simulink model "dss_ideal_8192ch_1966mhz_cx"  (R2021a) (continued)
== system dss_ideal_8192ch_1966mhz_cx == (continued)
      mask help: This simple vector accumulator is based on a shift register. It outputs its previous accumulated vector after a new_acc pulse is received.
      mask init:
      | simple_bram_vacc_init(gcb, ...
      |     'vec_len', vec_len, ...
      |     'arith_type', arith_type, ...
      |     'n_bits', n_bits, ...
      |     'bin_pt', bin_pt);
      mask TypeOptions arith_type: Signed | Unsigned
  simple_bram_vacc28 : Subsystem  AncestorBlock=casper_library_accumulators/simple_bram_vacc  mask(simple_bram_vacc){vec_len=1024, arith_type=Unsigned, n_bits=64, bin_pt=36}
      mask prompts: vec_len "Vector length:"; arith_type "Output type:"; n_bits "Bit width (output):"; bin_pt "Binary point (output):"
      mask help: This simple vector accumulator is based on a shift register. It outputs its previous accumulated vector after a new_acc pulse is received.
      mask init:
      | simple_bram_vacc_init(gcb, ...
      |     'vec_len', vec_len, ...
      |     'arith_type', arith_type, ...
      |     'n_bits', n_bits, ...
      |     'bin_pt', bin_pt);
      mask TypeOptions arith_type: Signed | Unsigned
  simple_bram_vacc29 : Subsystem  AncestorBlock=casper_library_accumulators/simple_bram_vacc  mask(simple_bram_vacc){vec_len=1024, arith_type=Unsigned, n_bits=64, bin_pt=36}
      mask prompts: vec_len "Vector length:"; arith_type "Output type:"; n_bits "Bit width (output):"; bin_pt "Binary point (output):"
      mask help: This simple vector accumulator is based on a shift register. It outputs its previous accumulated vector after a new_acc pulse is received.
      mask init:
      | simple_bram_vacc_init(gcb, ...
      |     'vec_len', vec_len, ...
      |     'arith_type', arith_type, ...
      |     'n_bits', n_bits, ...
      |     'bin_pt', bin_pt);
      mask TypeOptions arith_type: Signed | Unsigned
  simple_bram_vacc3 : Subsystem  AncestorBlock=casper_library_accumulators/simple_bram_vacc  mask(simple_bram_vacc){vec_len=1024, arith_type=Unsigned, n_bits=64, bin_pt=36}
      mask prompts: vec_len "Vector length:"; arith_type "Output type:"; n_bits "Bit width (output):"; bin_pt "Binary point (output):"
      mask help: This simple vector accumulator is based on a shift register. It outputs its previous accumulated vector after a new_acc pulse is received.
      mask init:
      | simple_bram_vacc_init(gcb, ...
      |     'vec_len', vec_len, ...
      |     'arith_type', arith_type, ...
      |     'n_bits', n_bits, ...
      |     'bin_pt', bin_pt);
      mask TypeOptions arith_type: Signed | Unsigned
  simple_bram_vacc30 : Subsystem  AncestorBlock=casper_library_accumulators/simple_bram_vacc  mask(simple_bram_vacc){vec_len=1024, arith_type=Unsigned, n_bits=64, bin_pt=36}
      mask prompts: vec_len "Vector length:"; arith_type "Output type:"; n_bits "Bit width (output):"; bin_pt "Binary point (output):"
      mask help: This simple vector accumulator is based on a shift register. It outputs its previous accumulated vector after a new_acc pulse is received.
      mask init:
      | simple_bram_vacc_init(gcb, ...
      |     'vec_len', vec_len, ...
      |     'arith_type', arith_type, ...
      |     'n_bits', n_bits, ...
      |     'bin_pt', bin_pt);
      mask TypeOptions arith_type: Signed | Unsigned
  simple_bram_vacc31 : Subsystem  AncestorBlock=casper_library_accumulators/simple_bram_vacc  mask(simple_bram_vacc){vec_len=1024, arith_type=Unsigned, n_bits=64, bin_pt=36}
      mask prompts: vec_len "Vector length:"; arith_type "Output type:"; n_bits "Bit width (output):"; bin_pt "Binary point (output):"
      mask help: This simple vector accumulator is based on a shift register. It outputs its previous accumulated vector after a new_acc pulse is received.
      mask init:
      | simple_bram_vacc_init(gcb, ...
      |     'vec_len', vec_len, ...
      |     'arith_type', arith_type, ...
      |     'n_bits', n_bits, ...
      |     'bin_pt', bin_pt);
      mask TypeOptions arith_type: Signed | Unsigned
  simple_bram_vacc32 : Subsystem  AncestorBlock=casper_library_accumulators/simple_bram_vacc  mask(simple_bram_vacc){vec_len=1024, arith_type=Unsigned, n_bits=64, bin_pt=36}
      mask prompts: vec_len "Vector length:"; arith_type "Output type:"; n_bits "Bit width (output):"; bin_pt "Binary point (output):"
      mask help: This simple vector accumulator is based on a shift register. It outputs its previous accumulated vector after a new_acc pulse is received.
      mask init:
      | simple_bram_vacc_init(gcb, ...
      |     'vec_len', vec_len, ...
      |     'arith_type', arith_type, ...
      |     'n_bits', n_bits, ...
      |     'bin_pt', bin_pt);
      mask TypeOptions arith_type: Signed | Unsigned
  simple_bram_vacc4 : Subsystem  AncestorBlock=casper_library_accumulators/simple_bram_vacc  mask(simple_bram_vacc){vec_len=1024, arith_type=Unsigned, n_bits=64, bin_pt=36}
      mask prompts: vec_len "Vector length:"; arith_type "Output type:"; n_bits "Bit width (output):"; bin_pt "Binary point (output):"
      mask help: This simple vector accumulator is based on a shift register. It outputs its previous accumulated vector after a new_acc pulse is received.
      mask init:
      | simple_bram_vacc_init(gcb, ...
      |     'vec_len', vec_len, ...
      |     'arith_type', arith_type, ...
      |     'n_bits', n_bits, ...
      |     'bin_pt', bin_pt);
      mask TypeOptions arith_type: Signed | Unsigned
  simple_bram_vacc5 : Subsystem  AncestorBlock=casper_library_accumulators/simple_bram_vacc  mask(simple_bram_vacc){vec_len=1024, arith_type=Unsigned, n_bits=64, bin_pt=36}
      mask prompts: vec_len "Vector length:"; arith_type "Output type:"; n_bits "Bit width (output):"; bin_pt "Binary point (output):"
      mask help: This simple vector accumulator is based on a shift register. It outputs its previous accumulated vector after a new_acc pulse is received.
      mask init:
      | simple_bram_vacc_init(gcb, ...
      |     'vec_len', vec_len, ...
      |     'arith_type', arith_type, ...
      |     'n_bits', n_bits, ...
      |     'bin_pt', bin_pt);
      mask TypeOptions arith_type: Signed | Unsigned
  simple_bram_vacc6 : Subsystem  AncestorBlock=casper_library_accumulators/simple_bram_vacc  mask(simple_bram_vacc){vec_len=1024, arith_type=Unsigned, n_bits=64, bin_pt=36}
      mask prompts: vec_len "Vector length:"; arith_type "Output type:"; n_bits "Bit width (output):"; bin_pt "Binary point (output):"
      mask help: This simple vector accumulator is based on a shift register. It outputs its previous accumulated vector after a new_acc pulse is received.
      mask init:
      | simple_bram_vacc_init(gcb, ...
      |     'vec_len', vec_len, ...
      |     'arith_type', arith_type, ...
      |     'n_bits', n_bits, ...
      |     'bin_pt', bin_pt);
      mask TypeOptions arith_type: Signed | Unsigned
  simple_bram_vacc7 : Subsystem  AncestorBlock=casper_library_accumulators/simple_bram_vacc  mask(simple_bram_vacc){vec_len=1024, arith_type=Unsigned, n_bits=64, bin_pt=36}
      mask prompts: vec_len "Vector length:"; arith_type "Output type:"; n_bits "Bit width (output):"; bin_pt "Binary point (output):"
      mask help: This simple vector accumulator is based on a shift register. It outputs its previous accumulated vector after a new_acc pulse is received.
      mask init:
      | simple_bram_vacc_init(gcb, ...
      |     'vec_len', vec_len, ...
      |     'arith_type', arith_type, ...
      |     'n_bits', n_bits, ...
      |     'bin_pt', bin_pt);
      mask TypeOptions arith_type: Signed | Unsigned
  simple_bram_vacc8 : Subsystem  AncestorBlock=casper_library_accumulators/simple_bram_vacc  mask(simple_bram_vacc){vec_len=1024, arith_type=Unsigned, n_bits=64, bin_pt=36}
      mask prompts: vec_len "Vector length:"; arith_type "Output type:"; n_bits "Bit width (output):"; bin_pt "Binary point (output):"
      mask help: This simple vector accumulator is based on a shift register. It outputs its previous accumulated vector after a new_acc pulse is received.
      mask init:
      | simple_bram_vacc_init(gcb, ...
      |     'vec_len', vec_len, ...
      |     'arith_type', arith_type, ...
      |     'n_bits', n_bits, ...
      |     'bin_pt', bin_pt);
      mask TypeOptions arith_type: Signed | Unsigned
  simple_bram_vacc9 : Subsystem  AncestorBlock=casper_library_accumulators/simple_bram_vacc  mask(simple_bram_vacc){vec_len=1024, arith_type=Unsigned, n_bits=64, bin_pt=36}
      mask prompts: vec_len "Vector length:"; arith_type "Output type:"; n_bits "Bit width (output):"; bin_pt "Binary point (output):"
      mask help: This simple vector accumulator is based on a shift register. It outputs its previous accumulated vector after a new_acc pulse is received.
      mask init:
      | simple_bram_vacc_init(gcb, ...
      |     'vec_len', vec_len, ...
      |     'arith_type', arith_type, ...
      |     'n_bits', n_bits, ...
      |     'bin_pt', bin_pt);
      mask TypeOptions arith_type: Signed | Unsigned
  spect_cnt2 : xbsIndex_r4/Counter  cnt_type=Free Running  cnt_to=Inf  operation=Up  start_count=0  cnt_by_val=1  arith_type=Unsigned  n_bits=10  bin_pt=0  load_pin=off  rst=on  en=on  explicit_period=on  period=1  use_behavioral_HDL=off  implementation=Fabric  use_rpm=off  dbl_ovrd=off  block_type=counter
  sync_gen : Subsystem  AncestorBlock=casper_library_misc/sync_gen  mask(sync_gen){sim_acc_len=1, fft_size=8192, fft_simult_inputs=8, pfb_fir_taps=4, reorder_vec=[2 2 10], scale=8192}
      mask prompts: sim_acc_len "Simulation Accumulation Length"; fft_size "FFT Size"; fft_simult_inputs "Simultaneous Inputs (FFT)"; pfb_fir_taps "Taps in pfb_fir (use 1 if no pfb_fir)"; reorder_vec "Reorder Orders (e.g. [2 2 2])"
      mask help:
      | This block computes the sync period based on system parameters as
      | described in Casper memo.  Detailed descritions of the block's
      | variables can be found in the 'Block Documentation' section of
      | the CASPER wiki.
      mask init:
      | sync_gen_init(gcb, ...
      |     'sim_acc_len', sim_acc_len, ...
      |     'fft_size', fft_size, ...
      |     'fft_simult_inputs', fft_simult_inputs,...
      |     'pfb_fir_taps', pfb_fir_taps, ...
      |     'reorder_vec', reorder_vec, ...
      |     'scale', scale);
      settings: AttributesFormatString=sim_sync_period=335544320
      mask Help:
      | SyncPeriod=LCM(reorder_vec)*(acc_num)*(pfb_fir_len)*(fft_size/fft_simult_inputs)
      | 
      | See CASPER sync pulse memo for more details.
  synth0_0 : Subsystem  Tag=xps:bram  AncestorBlock=xps_library/Memory/shared_bram  mask{arith_type=Unsigned, addr_width=10, data_width=64, reg_prim_output=on, reg_core_output=on, optimization=Minimum_Area, data_bin_pt=0, init_vals=[0:2^10-1], sample_rate=1}
      mask prompts: arith_type "Output Data Type"; addr_width "Address width"; data_width "Data Width"; reg_prim_output "Register Primitive Output"; reg_core_output "Register Core Output"; data_bin_pt "Data Binary Point"; init_vals "Initial values (simulation only)"; sample_rate "Sample rate"
      mask init:
      | shared_bram_mask;
      mask TypeOptions arith_type: Unsigned | Signed  (2's comp)
      mask TypeOptions data_width: 8 | 16 | 32 | 64 | 128
      mask TypeOptions optimization: Minimum_Area | Low_Power
  synth0_1 : Subsystem  Tag=xps:bram  AncestorBlock=xps_library/Memory/shared_bram  mask{arith_type=Unsigned, addr_width=10, data_width=64, reg_prim_output=on, reg_core_output=on, optimization=Minimum_Area, data_bin_pt=0, init_vals=[0:2^10-1], sample_rate=1}
      mask prompts: arith_type "Output Data Type"; addr_width "Address width"; data_width "Data Width"; reg_prim_output "Register Primitive Output"; reg_core_output "Register Core Output"; data_bin_pt "Data Binary Point"; init_vals "Initial values (simulation only)"; sample_rate "Sample rate"
      mask init:
      | shared_bram_mask;
      mask TypeOptions arith_type: Unsigned | Signed  (2's comp)
      mask TypeOptions data_width: 8 | 16 | 32 | 64 | 128
      mask TypeOptions optimization: Minimum_Area | Low_Power
  synth0_2 : Subsystem  Tag=xps:bram  AncestorBlock=xps_library/Memory/shared_bram  mask{arith_type=Unsigned, addr_width=10, data_width=64, reg_prim_output=on, reg_core_output=on, optimization=Minimum_Area, data_bin_pt=0, init_vals=[0:2^10-1], sample_rate=1}
      mask prompts: arith_type "Output Data Type"; addr_width "Address width"; data_width "Data Width"; reg_prim_output "Register Primitive Output"; reg_core_output "Register Core Output"; data_bin_pt "Data Binary Point"; init_vals "Initial values (simulation only)"; sample_rate "Sample rate"
      mask init:
      | shared_bram_mask;
      mask TypeOptions arith_type: Unsigned | Signed  (2's comp)
      mask TypeOptions data_width: 8 | 16 | 32 | 64 | 128
      mask TypeOptions optimization: Minimum_Area | Low_Power
  synth0_3 : Subsystem  Tag=xps:bram  AncestorBlock=xps_library/Memory/shared_bram  mask{arith_type=Unsigned, addr_width=10, data_width=64, reg_prim_output=on, reg_core_output=on, optimization=Minimum_Area, data_bin_pt=0, init_vals=[0:2^10-1], sample_rate=1}
      mask prompts: arith_type "Output Data Type"; addr_width "Address width"; data_width "Data Width"; reg_prim_output "Register Primitive Output"; reg_core_output "Register Core Output"; data_bin_pt "Data Binary Point"; init_vals "Initial values (simulation only)"; sample_rate "Sample rate"
      mask init:
      | shared_bram_mask;
      mask TypeOptions arith_type: Unsigned | Signed  (2's comp)
      mask TypeOptions data_width: 8 | 16 | 32 | 64 | 128
      mask TypeOptions optimization: Minimum_Area | Low_Power
  synth0_4 : Subsystem  Tag=xps:bram  AncestorBlock=xps_library/Memory/shared_bram  mask{arith_type=Unsigned, addr_width=10, data_width=64, reg_prim_output=on, reg_core_output=on, optimization=Minimum_Area, data_bin_pt=0, init_vals=[0:2^10-1], sample_rate=1}
      mask prompts: arith_type "Output Data Type"; addr_width "Address width"; data_width "Data Width"; reg_prim_output "Register Primitive Output"; reg_core_output "Register Core Output"; data_bin_pt "Data Binary Point"; init_vals "Initial values (simulation only)"; sample_rate "Sample rate"
      mask init:
      | shared_bram_mask;
      mask TypeOptions arith_type: Unsigned | Signed  (2's comp)
      mask TypeOptions data_width: 8 | 16 | 32 | 64 | 128
      mask TypeOptions optimization: Minimum_Area | Low_Power
  synth0_5 : Subsystem  Tag=xps:bram  AncestorBlock=xps_library/Memory/shared_bram  mask{arith_type=Unsigned, addr_width=10, data_width=64, reg_prim_output=on, reg_core_output=on, optimization=Minimum_Area, data_bin_pt=0, init_vals=[0:2^10-1], sample_rate=1}
      mask prompts: arith_type "Output Data Type"; addr_width "Address width"; data_width "Data Width"; reg_prim_output "Register Primitive Output"; reg_core_output "Register Core Output"; data_bin_pt "Data Binary Point"; init_vals "Initial values (simulation only)"; sample_rate "Sample rate"
      mask init:
      | shared_bram_mask;
      mask TypeOptions arith_type: Unsigned | Signed  (2's comp)
      mask TypeOptions data_width: 8 | 16 | 32 | 64 | 128
      mask TypeOptions optimization: Minimum_Area | Low_Power
  synth0_6 : Subsystem  Tag=xps:bram  AncestorBlock=xps_library/Memory/shared_bram  mask{arith_type=Unsigned, addr_width=10, data_width=64, reg_prim_output=on, reg_core_output=on, optimization=Minimum_Area, data_bin_pt=0, init_vals=[0:2^10-1], sample_rate=1}
      mask prompts: arith_type "Output Data Type"; addr_width "Address width"; data_width "Data Width"; reg_prim_output "Register Primitive Output"; reg_core_output "Register Core Output"; data_bin_pt "Data Binary Point"; init_vals "Initial values (simulation only)"; sample_rate "Sample rate"
      mask init:
      | shared_bram_mask;
      mask TypeOptions arith_type: Unsigned | Signed  (2's comp)
      mask TypeOptions data_width: 8 | 16 | 32 | 64 | 128
      mask TypeOptions optimization: Minimum_Area | Low_Power
  synth0_7 : Subsystem  Tag=xps:bram  AncestorBlock=xps_library/Memory/shared_bram  mask{arith_type=Unsigned, addr_width=10, data_width=64, reg_prim_output=on, reg_core_output=on, optimization=Minimum_Area, data_bin_pt=0, init_vals=[0:2^10-1], sample_rate=1}
      mask prompts: arith_type "Output Data Type"; addr_width "Address width"; data_width "Data Width"; reg_prim_output "Register Primitive Output"; reg_core_output "Register Core Output"; data_bin_pt "Data Binary Point"; init_vals "Initial values (simulation only)"; sample_rate "Sample rate"
      mask init:
      | shared_bram_mask;
      mask TypeOptions arith_type: Unsigned | Signed  (2's comp)
      mask TypeOptions data_width: 8 | 16 | 32 | 64 | 128
      mask TypeOptions optimization: Minimum_Area | Low_Power
  synth1_0 : Subsystem  Tag=xps:bram  AncestorBlock=xps_library/Memory/shared_bram  mask{arith_type=Unsigned, addr_width=10, data_width=64, reg_prim_output=on, reg_core_output=on, optimization=Minimum_Area, data_bin_pt=0, init_vals=[0:2^10-1], sample_rate=1}
      mask prompts: arith_type "Output Data Type"; addr_width "Address width"; data_width "Data Width"; reg_prim_output "Register Primitive Output"; reg_core_output "Register Core Output"; data_bin_pt "Data Binary Point"; init_vals "Initial values (simulation only)"; sample_rate "Sample rate"
      mask init:
      | shared_bram_mask;
      mask TypeOptions arith_type: Unsigned | Signed  (2's comp)
      mask TypeOptions data_width: 8 | 16 | 32 | 64 | 128
      mask TypeOptions optimization: Minimum_Area | Low_Power
  synth1_1 : Subsystem  Tag=xps:bram  AncestorBlock=xps_library/Memory/shared_bram  mask{arith_type=Unsigned, addr_width=10, data_width=64, reg_prim_output=on, reg_core_output=on, optimization=Minimum_Area, data_bin_pt=0, init_vals=[0:2^10-1], sample_rate=1}
      mask prompts: arith_type "Output Data Type"; addr_width "Address width"; data_width "Data Width"; reg_prim_output "Register Primitive Output"; reg_core_output "Register Core Output"; data_bin_pt "Data Binary Point"; init_vals "Initial values (simulation only)"; sample_rate "Sample rate"
      mask init:
      | shared_bram_mask;
      mask TypeOptions arith_type: Unsigned | Signed  (2's comp)
      mask TypeOptions data_width: 8 | 16 | 32 | 64 | 128
      mask TypeOptions optimization: Minimum_Area | Low_Power
  synth1_2 : Subsystem  Tag=xps:bram  AncestorBlock=xps_library/Memory/shared_bram  mask{arith_type=Unsigned, addr_width=10, data_width=64, reg_prim_output=on, reg_core_output=on, optimization=Minimum_Area, data_bin_pt=0, init_vals=[0:2^10-1], sample_rate=1}
      mask prompts: arith_type "Output Data Type"; addr_width "Address width"; data_width "Data Width"; reg_prim_output "Register Primitive Output"; reg_core_output "Register Core Output"; data_bin_pt "Data Binary Point"; init_vals "Initial values (simulation only)"; sample_rate "Sample rate"
      mask init:
      | shared_bram_mask;
      mask TypeOptions arith_type: Unsigned | Signed  (2's comp)
      mask TypeOptions data_width: 8 | 16 | 32 | 64 | 128
      mask TypeOptions optimization: Minimum_Area | Low_Power
  synth1_3 : Subsystem  Tag=xps:bram  AncestorBlock=xps_library/Memory/shared_bram  mask{arith_type=Unsigned, addr_width=10, data_width=64, reg_prim_output=on, reg_core_output=on, optimization=Minimum_Area, data_bin_pt=0, init_vals=[0:2^10-1], sample_rate=1}
      mask prompts: arith_type "Output Data Type"; addr_width "Address width"; data_width "Data Width"; reg_prim_output "Register Primitive Output"; reg_core_output "Register Core Output"; data_bin_pt "Data Binary Point"; init_vals "Initial values (simulation only)"; sample_rate "Sample rate"
      mask init:
      | shared_bram_mask;
      mask TypeOptions arith_type: Unsigned | Signed  (2's comp)
      mask TypeOptions data_width: 8 | 16 | 32 | 64 | 128
      mask TypeOptions optimization: Minimum_Area | Low_Power
  synth1_4 : Subsystem  Tag=xps:bram  AncestorBlock=xps_library/Memory/shared_bram  mask{arith_type=Unsigned, addr_width=10, data_width=64, reg_prim_output=on, reg_core_output=on, optimization=Minimum_Area, data_bin_pt=0, init_vals=[0:2^10-1], sample_rate=1}
      mask prompts: arith_type "Output Data Type"; addr_width "Address width"; data_width "Data Width"; reg_prim_output "Register Primitive Output"; reg_core_output "Register Core Output"; data_bin_pt "Data Binary Point"; init_vals "Initial values (simulation only)"; sample_rate "Sample rate"
      mask init:
      | shared_bram_mask;
      mask TypeOptions arith_type: Unsigned | Signed  (2's comp)
      mask TypeOptions data_width: 8 | 16 | 32 | 64 | 128
      mask TypeOptions optimization: Minimum_Area | Low_Power
  synth1_5 : Subsystem  Tag=xps:bram  AncestorBlock=xps_library/Memory/shared_bram  mask{arith_type=Unsigned, addr_width=10, data_width=64, reg_prim_output=on, reg_core_output=on, optimization=Minimum_Area, data_bin_pt=0, init_vals=[0:2^10-1], sample_rate=1}
      mask prompts: arith_type "Output Data Type"; addr_width "Address width"; data_width "Data Width"; reg_prim_output "Register Primitive Output"; reg_core_output "Register Core Output"; data_bin_pt "Data Binary Point"; init_vals "Initial values (simulation only)"; sample_rate "Sample rate"
      mask init:
      | shared_bram_mask;
      mask TypeOptions arith_type: Unsigned | Signed  (2's comp)
      mask TypeOptions data_width: 8 | 16 | 32 | 64 | 128
      mask TypeOptions optimization: Minimum_Area | Low_Power
  synth1_6 : Subsystem  Tag=xps:bram  AncestorBlock=xps_library/Memory/shared_bram  mask{arith_type=Unsigned, addr_width=10, data_width=64, reg_prim_output=on, reg_core_output=on, optimization=Minimum_Area, data_bin_pt=0, init_vals=[0:2^10-1], sample_rate=1}
      mask prompts: arith_type "Output Data Type"; addr_width "Address width"; data_width "Data Width"; reg_prim_output "Register Primitive Output"; reg_core_output "Register Core Output"; data_bin_pt "Data Binary Point"; init_vals "Initial values (simulation only)"; sample_rate "Sample rate"
      mask init:
      | shared_bram_mask;
      mask TypeOptions arith_type: Unsigned | Signed  (2's comp)
      mask TypeOptions data_width: 8 | 16 | 32 | 64 | 128
      mask TypeOptions optimization: Minimum_Area | Low_Power
  synth1_7 : Subsystem  Tag=xps:bram  AncestorBlock=xps_library/Memory/shared_bram  mask{arith_type=Unsigned, addr_width=10, data_width=64, reg_prim_output=on, reg_core_output=on, optimization=Minimum_Area, data_bin_pt=0, init_vals=[0:2^10-1], sample_rate=1}
      mask prompts: arith_type "Output Data Type"; addr_width "Address width"; data_width "Data Width"; reg_prim_output "Register Primitive Output"; reg_core_output "Register Core Output"; data_bin_pt "Data Binary Point"; init_vals "Initial values (simulation only)"; sample_rate "Sample rate"
      mask init:
      | shared_bram_mask;
      mask TypeOptions arith_type: Unsigned | Signed  (2's comp)
      mask TypeOptions data_width: 8 | 16 | 32 | 64 | 128
      mask TypeOptions optimization: Minimum_Area | Low_Power
  wires:
  rfdc/1(m00_axis_tdata) -> bus_create/1(in1)
  rfdc/2(m01_axis_tdata) -> bus_create/2(in2)
  bus_create/1(bus_out) -> munge1/1(din), bus_expand2/1(bus_in)
  bus_expand/8(lsb_out1) -> pfb_fir/9(pol1_in8)
  bus_expand/1(msb_out8) -> pfb_fir/2(pol1_in1)
  bus_expand/3(out6) -> pfb_fir/4(pol1_in3)
  pfb_fir/7(pol1_out6) -> fft/8(in05)
  pfb_fir/1(sync_out) -> fft/1(sync)
  pfb_fir/9(pol1_out8) -> fft/10(in07)
  bus_expand/4(out5) -> pfb_fir/5(pol1_in4)
  munge1/1(dout) -> bus_expand/1(bus_in)
  pipeline3/1(q) -> pfb_fir/1(sync)
  pfb_fir/5(pol1_out4) -> fft/6(in03)
  Constant2/1 -> fft/2(shift)
  bus_expand/7(out2) -> pfb_fir/8(pol1_in7)
  bus_expand/5(out4) -> pfb_fir/6(pol1_in5)
  bus_expand/6(out3) -> pfb_fir/7(pol1_in6)
  pfb_fir/6(pol1_out5) -> fft/7(in04)
  bus_expand/2(out7) -> pfb_fir/3(pol1_in2)
  pfb_fir/4(pol1_out3) -> fft/5(in02)
  pfb_fir/2(pol1_out1) -> fft/3(in00)
  pfb_fir/8(pol1_out7) -> fft/9(in06)
  pfb_fir/3(pol1_out2) -> fft/4(in01)
  [sync_gen] -> Delay23/1
  Delay23/1 -> pipeline3/1(d)
  Band-Limited White Noise/1 -> rfdc/10(m23_axis_tdata_sim), rfdc/11(m30_axis_tdata_sim), rfdc/12(m32_axis_tdata_sim), rfdc/9(m22_axis_tdata_sim), rfdc/8(m21_axis_tdata_sim), rfdc/7(m20_axis_tdata_sim), rfdc/6(m12_axis_tdata_sim), rfdc/5(m10_axis_tdata_sim), rfdc/4(m03_axis_tdata_sim), rfdc/3(m02_axis_tdata_sim), rfdc/2(m01_axis_tdata_sim), rfdc/1(m00_axis_tdata_sim)
  rfdc/5(m10_axis_tdata) -> Terminator4/1
  rfdc/6(m12_axis_tdata) -> Terminator9/1
  rfdc/8(m21_axis_tdata) -> Terminator17/1
  rfdc/12(m32_axis_tdata) -> Terminator19/1
  rfdc/7(m20_axis_tdata) -> Terminator16/1
  rfdc/9(m22_axis_tdata) -> Terminator20/1
  rfdc/10(m23_axis_tdata) -> Terminator21/1
  rfdc/11(m30_axis_tdata) -> Terminator18/1
  pipeline157/1(q) -> comp_add/1(a)
  pipeline113/1(q) -> spect_cnt2/1
  simple_bram_vacc1/2(valid) -> spect_cnt2/2, pipeline118/1(d)
  pipeline121/1(q) -> pipeline126/1(d)
  pipeline161/1(q) -> Delay46/1
  pipeline126/1(q) -> simple_bram_vacc1/2(din)
  bram_mult0_0/1(c) -> comp_add/2(b)
  Delay46/1 -> Delay43/1
  Reinterpret21/1 -> pipeline117/1(d)
  simple_bram_vacc1/1(dout) -> Reinterpret21/1
  pipeline118/1(q) -> synth0_0/3(we)
  pipeline162/1(q) -> pipeline161/1(d)
  synth0_0/1(data_out) -> Terminator1/1
  pipeline125/1(q) -> simple_bram_vacc1/1(new_acc)
  comp_add/1(a+b) -> power1/1
  Delay44/1 -> pipeline121/1(d)
  pipeline104/1(q) -> synth0_0/1(addr)
  [acc_len] -> acc_cntrl/1(acc_len)
  Delay43/1 -> acc_cntrl/2(sync)
  [cnt_rst] -> acc_cntrl/3(rst)
  pipeline117/1(q) -> synth0_0/2(data_in)
  power1/1 -> Delay44/1
  fft/1(sync_out) -> Delay39/1
  fft/10(of) -> Terminator2/1
  Delay39/1 -> Delay38/1
  Delay38/1 -> pipeline162/1(d), bram_mult0_1/1(In1), bram_mult0_2/1(In1), bram_mult0_3/1(In1), bram_mult0_4/1(In1), bram_mult0_5/1(In1), bram_mult0_8/1(In1), bram_mult0_9/1(In1), bram_mult0_10/1(In1), bram_mult0_11/1(In1), bram_mult0_12/1(In1), bram_mult0_13/1(In1), bram_mult0_14/1(In1), bram_mult0_15/1(In1), bram_mult0_7/1(In1), bram_mult0_6/1(In1), bram_mult0_0/1(In1)
  Delay15/1 -> bram_mult0_8/2(d), cmult/1(a), pipeline157/1(d)
  fft/2(out00) -> Delay18/1
  Delay18/1 -> Delay15/1
  Delay3/1 -> bram_mult0_9/2(d), cmult1/1(a), pipeline10/1(d)
  fft/3(out01) -> Delay4/1
  Delay4/1 -> Delay3/1
  Delay2/1 -> Delay1/1
  fft/4(out02) -> Delay2/1
  Delay1/1 -> bram_mult0_10/2(d), cmult2/1(a), pipeline12/1(d)
  fft/6(out04) -> Delay6/1
  Delay8/1 -> Delay7/1
  Delay6/1 -> Delay5/1
  fft/5(out03) -> Delay8/1
  Delay5/1 -> bram_mult0_12/2(d), cmult4/1(a), pipeline21/1(d)
  Delay7/1 -> bram_mult0_11/2(d), cmult3/1(a), pipeline19/1(d)
  Delay10/1 -> Delay9/1
  fft/9(out07) -> Delay12/1
  fft/8(out06) -> Delay14/1
  Delay12/1 -> Delay11/1
  Delay13/1 -> bram_mult0_14/2(d), cmult6/1(a), pipeline40/1(d)
  fft/7(out05) -> Delay10/1
  Delay11/1 -> bram_mult0_15/2(d), cmult7/1(a), pipeline42/1(d)
  Delay9/1 -> bram_mult0_13/2(d), cmult5/1(a), pipeline33/1(d)
  Delay14/1 -> Delay13/1
  pipeline1/1(q) -> synth0_1/1(addr)
  pipeline4/1(q) -> synth0_1/3(we)
  simple_bram_vacc2/1(dout) -> Reinterpret1/1
  pipeline2/1(q) -> synth0_1/2(data_in)
  pipeline6/1(q) -> simple_bram_vacc2/1(new_acc)
  bram_mult0_1/1(c) -> comp_add1/2(b)
  Delay16/1 -> pipeline5/1(d)
  pipeline10/1(q) -> comp_add1/1(a)
  power2/1 -> Delay16/1
  pipeline5/1(q) -> pipeline7/1(d)
  synth0_1/1(data_out) -> Terminator3/1
  simple_bram_vacc2/2(valid) -> pipeline4/1(d)
  comp_add1/1(a+b) -> power2/1
  pipeline7/1(q) -> simple_bram_vacc2/2(din)
  Reinterpret1/1 -> pipeline2/1(d)
  Delay17/1 -> pipeline15/1(d)
  Reinterpret2/1 -> pipeline13/1(d)
  pipeline12/1(q) -> comp_add2/1(a)
  pipeline15/1(q) -> pipeline17/1(d)
  simple_bram_vacc3/2(valid) -> pipeline14/1(d)
  power3/1 -> Delay17/1
  bram_mult0_2/1(c) -> comp_add2/2(b)
  synth0_2/1(data_out) -> Terminator5/1
  comp_add2/1(a+b) -> power3/1
  pipeline17/1(q) -> simple_bram_vacc3/2(din)
  pipeline13/1(q) -> synth0_2/2(data_in)
  simple_bram_vacc3/1(dout) -> Reinterpret2/1
  pipeline16/1(q) -> simple_bram_vacc3/1(new_acc)
  pipeline14/1(q) -> synth0_2/3(we)
  Delay19/1 -> pipeline29/1(d)
  bram_mult0_4/1(c) -> comp_add4/2(b)
  pipeline27/1(q) -> synth0_3/2(data_in)
  comp_add4/1(a+b) -> power5/1
  pipeline31/1(q) -> simple_bram_vacc4/2(din)
  pipeline23/1(q) -> synth0_4/3(we)
  bram_mult0_3/1(c) -> comp_add3/2(b)
  Reinterpret3/1 -> pipeline27/1(d)
  pipeline29/1(q) -> pipeline31/1(d)
  pipeline19/1(q) -> comp_add3/1(a)
  power5/1 -> Delay20/1
  Reinterpret4/1 -> pipeline22/1(d)
  pipeline22/1(q) -> synth0_4/2(data_in)
  pipeline24/1(q) -> pipeline26/1(d)
  pipeline25/1(q) -> simple_bram_vacc5/1(new_acc)
  Delay20/1 -> pipeline24/1(d)
  simple_bram_vacc4/2(valid) -> pipeline28/1(d)
  simple_bram_vacc5/2(valid) -> pipeline23/1(d)
  synth0_3/1(data_out) -> Terminator6/1
  synth0_4/1(data_out) -> Terminator7/1
  simple_bram_vacc4/1(dout) -> Reinterpret3/1
  pipeline30/1(q) -> simple_bram_vacc4/1(new_acc)
  power4/1 -> Delay19/1
  comp_add3/1(a+b) -> power4/1
  pipeline21/1(q) -> comp_add4/1(a)
  simple_bram_vacc5/1(dout) -> Reinterpret4/1
  pipeline26/1(q) -> simple_bram_vacc5/2(din)
  pipeline28/1(q) -> synth0_3/3(we)
  pipeline47/1(q) -> simple_bram_vacc8/2(din)
  pipeline51/1(q) -> simple_bram_vacc7/1(new_acc)
  simple_bram_vacc6/2(valid) -> pipeline35/1(d)
  pipeline33/1(q) -> comp_add5/1(a)
  simple_bram_vacc6/1(dout) -> Reinterpret5/1
  pipeline43/1(q) -> synth0_7/2(data_in)
  synth0_6/1(data_out) -> Terminator10/1
  power8/1 -> Delay24/1
  Delay22/1 -> pipeline50/1(d)
  power7/1 -> Delay22/1
  simple_bram_vacc8/2(valid) -> pipeline44/1(d)
  Delay21/1 -> pipeline36/1(d)
  simple_bram_vacc8/1(dout) -> Reinterpret7/1
  Reinterpret5/1 -> pipeline34/1(d)
  bram_mult0_5/1(c) -> comp_add5/2(b)
  pipeline45/1(q) -> pipeline47/1(d)
  comp_add6/1(a+b) -> power7/1
  pipeline48/1(q) -> synth0_6/2(data_in)
  simple_bram_vacc7/1(dout) -> Reinterpret6/1
  simple_bram_vacc7/2(valid) -> pipeline49/1(d)
  bram_mult0_7/1(c) -> comp_add7/2(b)
  pipeline44/1(q) -> synth0_7/3(we)
  pipeline34/1(q) -> synth0_5/2(data_in)
  synth0_7/1(data_out) -> Terminator11/1
  pipeline42/1(q) -> comp_add7/1(a)
  bram_mult0_6/1(c) -> comp_add6/2(b)
  pipeline52/1(q) -> simple_bram_vacc7/2(din)
  Reinterpret6/1 -> pipeline48/1(d)
  Delay24/1 -> pipeline45/1(d)
  synth0_5/1(data_out) -> Terminator8/1
  pipeline37/1(q) -> simple_bram_vacc6/1(new_acc)
  pipeline36/1(q) -> pipeline38/1(d)
  pipeline50/1(q) -> pipeline52/1(d)
  pipeline46/1(q) -> simple_bram_vacc8/1(new_acc)
  Reinterpret7/1 -> pipeline43/1(d)
  pipeline35/1(q) -> synth0_5/3(we)
  comp_add5/1(a+b) -> power6/1
  pipeline49/1(q) -> synth0_6/3(we)
  comp_add7/1(a+b) -> power8/1
  pipeline38/1(q) -> simple_bram_vacc6/2(din)
  pipeline40/1(q) -> comp_add6/1(a)
  power6/1 -> Delay21/1
  Delay57/1 -> Delay56/1
  simple_bram_vacc13/1(dout) -> Reinterpret12/1
  pipeline76/1(q) -> comp_add12/1(a)
  fft1/7(out05) -> Delay26/1
  Delay55/1 -> Delay54/1
  pipeline99/1(q) -> comp_add15/1(a)
  bus_expand1/2(out7) -> pfb_fir1/3(pol1_in2)
  synth1_5/1(data_out) -> Terminator25/1
  bram_mult0_14/1(c) -> comp_add14/2(b)
  power13/1 -> Delay37/1
  simple_bram_vacc16/1(dout) -> Reinterpret15/1
  fft1/8(out06) -> Delay30/1
  pipeline94/1(q) -> simple_bram_vacc14/2(din)
  fft1/3(out01) -> Delay50/1
  Delay35/1 -> pipeline84/1(d)
  pipeline55/1(q) -> synth1_0/1(addr)
  pipeline80/1(q) -> simple_bram_vacc13/1(new_acc)
  pipeline89/1(q) -> comp_add13/1(a)
  Delay58/1 -> bram_mult0_5/2(d), cmult5/2(b), pipeline89/1(d)
  pipeline86/1(q) -> simple_bram_vacc12/1(new_acc)
  Delay54/1 -> bram_mult0_4/2(d), cmult4/2(b), pipeline76/1(d)
  pipeline97/1(q) -> comp_add14/1(a)
  simple_bram_vacc9/1(dout) -> Reinterpret10/1
  simple_bram_vacc10/1(dout) -> Reinterpret8/1
  pfb_fir1/3(pol1_out2) -> fft1/4(in01)
  Constant1/1 -> fft1/2(shift)
  Delay34/1 -> Delay31/1
  power14/1 -> Delay40/1
  simple_bram_vacc14/2(valid) -> pipeline91/1(d)
  simple_bram_vacc15/1(dout) -> Reinterpret14/1
  simple_bram_vacc9/2(valid) -> pipeline59/1(d)
  Delay41/1 -> pipeline109/1(d)
  simple_bram_vacc12/2(valid) -> pipeline83/1(d)
  Delay52/1 -> pipeline61/1(d)
  power15/1 -> Delay41/1
  Reinterpret13/1 -> pipeline90/1(d)
  Delay33/1 -> pipeline66/1(d)
  fft1/2(out00) -> Delay34/1
  bus_expand1/8(lsb_out1) -> pfb_fir1/9(pol1_in8)
  simple_bram_vacc13/2(valid) -> pipeline78/1(d)
  pipeline62/1(q) -> simple_bram_vacc9/1(new_acc)
  bram_mult0_11/1(c) -> comp_add11/2(b)
  Reinterpret9/1 -> pipeline64/1(d)
  Delay37/1 -> pipeline79/1(d)
  pipeline105/1(q) -> simple_bram_vacc16/2(din)
  pipeline87/1(q) -> simple_bram_vacc12/2(din)
  simple_bram_vacc16/2(valid) -> pipeline101/1(d)
  pfb_fir1/4(pol1_out3) -> fft1/5(in02)
  pipeline92/1(q) -> pipeline94/1(d)
  pipeline68/1(q) -> simple_bram_vacc11/1(new_acc)
  power12/1 -> Delay35/1
  pfb_fir1/9(pol1_out8) -> fft1/10(in07)
  fft1/4(out02) -> Delay36/1
  pipeline65/1(q) -> synth1_2/3(we)
  pipeline91/1(q) -> synth1_5/3(we)
  Reinterpret8/1 -> pipeline74/1(d)
  bram_mult0_12/1(c) -> comp_add12/2(b)
  bus_expand1/3(out6) -> pfb_fir1/4(pol1_in3)
  fft1/6(out04) -> Delay55/1
  pipeline90/1(q) -> synth1_5/2(data_in)
  pipeline107/1(q) -> synth1_6/3(we)
  fft1/9(out07) -> Delay28/1
  synth1_1/1(data_out) -> Terminator15/1
  pfb_fir1/6(pol1_out5) -> fft1/7(in04)
  bram_mult0_10/1(c) -> comp_add10/2(b)
  pipeline79/1(q) -> pipeline81/1(d)
  pipeline106/1(q) -> synth1_6/2(data_in)
  pipeline63/1(q) -> simple_bram_vacc9/2(din)
  Reinterpret14/1 -> pipeline106/1(d)
  pipeline71/1(q) -> simple_bram_vacc11/2(din)
  simple_bram_vacc11/2(valid) -> pipeline65/1(d)
  Delay56/1 -> cmult3/2(b), bram_mult0_3/2(d), pipeline73/1(d)
  pipeline59/1(q) -> synth1_0/3(we)
  pipeline78/1(q) -> synth1_4/3(we)
  bram_mult0_8/1(c) -> comp_add8/2(b)
  simple_bram_vacc15/2(valid) -> pipeline107/1(d)
  pfb_fir1/5(pol1_out4) -> fft1/6(in03)
  pipeline85/1(q) -> pfb_fir1/1(sync)
  pfb_fir1/1(sync_out) -> fft1/1(sync)
  bram_mult0_13/1(c) -> comp_add13/2(b)
  pipeline77/1(q) -> synth1_4/2(data_in)
  bus_expand1/5(out4) -> pfb_fir1/6(pol1_in5)
  bus_expand1/1(msb_out8) -> pfb_fir1/2(pol1_in1)
  Delay29/1 -> bram_mult0_6/2(d), cmult6/2(b), pipeline97/1(d)
  spect_cnt2/1 -> pipeline104/1(d), pipeline53/1(d), pipeline56/1(d), pipeline72/1(d), pipeline75/1(d), pipeline88/1(d), pipeline95/1(d), pipeline120/1(d), pipeline139/1(d), pipeline122/1(d), pipeline119/1(d), pipeline156/1(d), pipeline174/1(d), pipeline198/1(d), pipeline184/1(d), pipeline190/1(d), pipeline173/1(d), pipeline207/1(d), pipeline213/1(d), pipeline175/1(d), pipeline165/1(d), pipeline133/1(d), pipeline147/1(d), pipeline98/1(d), pipeline55/1(d), pipeline41/1(d), pipeline39/1(d), pipeline32/1(d), pipeline20/1(d), pipeline18/1(d), pipeline11/1(d), pipeline1/1(d)
  pipeline108/1(q) -> pipeline114/1(d)
  Delay27/1 -> bram_mult0_7/2(d), cmult7/2(b), pipeline99/1(d)
  Delay50/1 -> Delay47/1
  Reinterpret11/1 -> pipeline82/1(d)
  synth1_7/1(data_out) -> Terminator14/1
  Delay26/1 -> Delay58/1
  pipeline66/1(q) -> pipeline71/1(d)
  Reinterpret15/1 -> pipeline100/1(d)
  fft1/10(of) -> Terminator/1
  pipeline82/1(q) -> synth1_3/2(data_in)
  power9/1 -> Delay52/1
  simple_bram_vacc14/1(dout) -> Reinterpret13/1
  pipeline54/1(q) -> comp_add9/1(a)
  Reinterpret10/1 -> pipeline58/1(d)
  pipeline64/1(q) -> synth1_2/2(data_in)
  power10/1 -> Delay32/1
  Delay47/1 -> bram_mult0_1/2(d), cmult1/2(b), pipeline54/1(d)
  pipeline101/1(q) -> synth1_7/3(we)
  Delay42/1 -> pipeline85/1(d)
  bram_mult0_15/1(c) -> comp_add15/2(b)
  pipeline84/1(q) -> pipeline87/1(d)
  simple_bram_vacc12/1(dout) -> Reinterpret11/1
  power11/1 -> Delay33/1
  [sync_gen] -> Delay42/1
  fft1/5(out03) -> Delay57/1
  Delay36/1 -> Delay25/1
  fft1/1(sync_out) -> Terminator80/1
  Reinterpret12/1 -> pipeline77/1(d)
  synth1_2/1(data_out) -> Terminator22/1
  pipeline93/1(q) -> simple_bram_vacc14/1(new_acc)
  Delay30/1 -> Delay29/1
  simple_bram_vacc10/2(valid) -> pipeline96/1(d)
  pipeline109/1(q) -> pipeline111/1(d)
  Delay45/1 -> pipeline102/1(d)
  pipeline67/1(q) -> comp_add8/1(a)
  Delay32/1 -> pipeline108/1(d)
  Delay40/1 -> pipeline92/1(d)
  bus_expand1/7(out2) -> pfb_fir1/8(pol1_in7)
  synth1_6/1(data_out) -> Terminator13/1
  pipeline61/1(q) -> pipeline63/1(d)
  pipeline58/1(q) -> synth1_0/2(data_in)
  pipeline100/1(q) -> synth1_7/2(data_in)
  synth1_3/1(data_out) -> Terminator23/1
  pipeline53/1(q) -> synth1_1/1(addr)
  pipeline60/1(q) -> comp_add10/1(a)
  pipeline102/1(q) -> pipeline105/1(d)
  power16/1 -> Delay45/1
  pipeline114/1(q) -> simple_bram_vacc10/2(din)
  bus_expand1/6(out3) -> pfb_fir1/7(pol1_in6)
  munge2/1(dout) -> bus_expand1/1(bus_in)
  Delay31/1 -> cmult/2(b), pipeline67/1(d), bram_mult0_0/2(d)
  pipeline81/1(q) -> simple_bram_vacc13/2(din)
  bram_mult0_9/1(c) -> comp_add9/2(b)
  simple_bram_vacc11/1(dout) -> Reinterpret9/1
  pipeline83/1(q) -> synth1_3/3(we)
  pipeline112/1(q) -> simple_bram_vacc10/1(new_acc)
  synth1_4/1(data_out) -> Terminator24/1
  Delay28/1 -> Delay27/1
  pipeline103/1(q) -> simple_bram_vacc16/1(new_acc)
  Delay25/1 -> bram_mult0_2/2(d), cmult2/2(b), pipeline60/1(d)
  pfb_fir1/2(pol1_out1) -> fft1/3(in00)
  pipeline74/1(q) -> synth1_1/2(data_in)
  pipeline73/1(q) -> comp_add11/1(a)
  pfb_fir1/8(pol1_out7) -> fft1/9(in06)
  pipeline111/1(q) -> simple_bram_vacc15/2(din)
  pfb_fir1/7(pol1_out6) -> fft1/8(in05)
  pipeline110/1(q) -> simple_bram_vacc15/1(new_acc)
  pipeline96/1(q) -> synth1_1/3(we)
  bus_expand1/4(out5) -> pfb_fir1/5(pol1_in4)
  synth1_0/1(data_out) -> Terminator12/1
  rfdc/3(m02_axis_tdata) -> bus_create1/1(in1)
  rfdc/4(m03_axis_tdata) -> bus_create1/2(in2)
  pipeline11/1(q) -> synth0_2/1(addr)
  pipeline18/1(q) -> synth0_3/1(addr)
  pipeline20/1(q) -> synth0_4/1(addr)
  pipeline32/1(q) -> synth0_5/1(addr)
  pipeline39/1(q) -> synth0_6/1(addr)
  pipeline41/1(q) -> synth0_7/1(addr)
  pipeline56/1(q) -> synth1_2/1(addr)
  pipeline72/1(q) -> synth1_3/1(addr)
  pipeline75/1(q) -> synth1_4/1(addr)
  pipeline88/1(q) -> synth1_5/1(addr)
  pipeline95/1(q) -> synth1_6/1(addr)
  pipeline98/1(q) -> synth1_7/1(addr)
  adc1_snapshot0/1(arm_out) -> Terminator28/1
  Reinterpret16/1 -> adc1_snapshot1/1(in_hi)
  Reinterpret17/1 -> adc1_snapshot0/1(in_hi)
  bus_expand2/2(lsb_out1) -> Reinterpret16/1
  adc1_snapshot1/1(arm_out) -> Terminator29/1
  bus_expand2/1(msb_out2) -> Reinterpret17/1
  Constant4/1 -> adc1_snapshot1/2(we), adc1_snapshot1/3(trig)
  Constant3/1 -> adc1_snapshot0/2(we), adc1_snapshot0/3(trig)
  Reinterpret18/1 -> adc1_snapshot3/1(in_hi)
  bus_expand3/2(lsb_out1) -> Reinterpret18/1
  adc1_snapshot2/1(arm_out) -> Terminator30/1
  bus_create1/1(bus_out) -> bus_expand3/1(bus_in), munge2/1(din)
  bus_expand3/1(msb_out2) -> Reinterpret19/1
  Reinterpret19/1 -> adc1_snapshot2/1(in_hi)
  Constant5/1 -> adc1_snapshot2/2(we), adc1_snapshot2/3(trig)
  Constant6/1 -> adc1_snapshot3/2(we), adc1_snapshot3/3(trig)
  adc1_snapshot3/1(arm_out) -> Terminator31/1
  comp_add9/1(a+b) -> power10/1
  comp_add8/1(a+b) -> power9/1
  comp_add10/1(a+b) -> power11/1
  comp_add11/1(a+b) -> power12/1
  comp_add12/1(a+b) -> power13/1
  comp_add13/1(a+b) -> power14/1
  comp_add14/1(a+b) -> power15/1
  comp_add15/1(a+b) -> power16/1
  pipeline165/1(q) -> ab_im3/1(addr)
  Reinterpret23/1 -> pipeline137/1(d)
  Delay63/1 -> pipeline143/1(d)
  simple_bram_vacc22/2(valid) -> pipeline153/1(d)
  pipeline153/1(q) -> ab_im2/3(we)
  pipeline147/1(q) -> ab_im0/1(addr)
  Reinterpret25/1 -> pipeline154/1(d)
  simple_bram_vacc20/1(dout) -> Reinterpret22/1
  pipeline170/1(q) -> pipeline151/1(d)
  pipeline130/1(q) -> ab_im0/3(we)
  ab_re0/1(data_out) -> Terminator26/1
  simple_bram_vacc22/1(dout) -> Reinterpret28/1
  pipeline132/1(q) -> ab_re1/3(we)
  Reinterpret20/1 -> pipeline131/1(d)
  cmult3/1(ab) -> c_to_ri3/1
  pipeline122/1(q) -> ab_re2/1(addr)
  simple_bram_vacc21/1(dout) -> Reinterpret27/1
  Reinterpret24/1 -> pipeline129/1(d)
  pipeline152/1(q) -> ab_im2/2(data_in)
  pipeline169/1(q) -> pipeline166/1(d)
  pipeline141/1(q) -> pipeline146/1(d)
  pipeline142/1(q) -> pipeline124/1(d)
  ab_im1/1(data_out) -> Terminator33/1
  pipeline143/1(q) -> pipeline140/1(d)
  pipeline167/1(q) -> pipeline116/1(d)
  Reinterpret28/1 -> pipeline152/1(d)
  Delay61/1 -> pipeline142/1(d)
  pipeline164/1(q) -> ab_re2/3(we)
  simple_bram_vacc18/2(valid) -> pipeline130/1(d)
  pipeline134/1(q) -> ab_im1/2(data_in)
  cmult1/1(ab) -> c_to_ri1/1
  simple_bram_vacc21/2(valid) -> pipeline164/1(d)
  pipeline139/1(q) -> ab_im1/1(addr)
  simple_bram_vacc19/1(dout) -> Reinterpret20/1
  Delay64/1 -> pipeline144/1(d)
  ab_re3/1(data_out) -> Terminator36/1
  Delay66/1 -> pipeline170/1(d)
  ab_im3/1(data_out) -> Terminator37/1
  pipeline158/1(q) -> ab_im3/2(data_in)
  Delay59/1 -> pipeline167/1(d)
  ab_im0/1(data_out) -> Terminator27/1
  ab_re1/1(data_out) -> Terminator32/1
  Reinterpret22/1 -> pipeline134/1(d)
  pipeline168/1(q) -> pipeline149/1(d)
  pipeline146/1(q) -> simple_bram_vacc17/2(din)
  simple_bram_vacc20/2(valid) -> pipeline136/1(d)
  simple_bram_vacc24/1(dout) -> Reinterpret26/1
  pipeline137/1(q) -> ab_re0/2(data_in)
  Delay60/1 -> pipeline141/1(d)
  Delay65/1 -> pipeline169/1(d)
  pipeline160/1(q) -> ab_im3/3(we)
  cmult/1(ab) -> c_to_ri/1
  pipeline131/1(q) -> ab_re1/2(data_in)
  pipeline129/1(q) -> ab_im0/2(data_in)
  ab_re2/1(data_out) -> Terminator34/1
  simple_bram_vacc24/2(valid) -> pipeline160/1(d)
  simple_bram_vacc23/2(valid) -> pipeline155/1(d)
  ab_im2/1(data_out) -> Terminator35/1
  pipeline119/1(q) -> ab_im2/1(addr)
  Reinterpret27/1 -> pipeline163/1(d)
  Reinterpret26/1 -> pipeline158/1(d)
  simple_bram_vacc19/2(valid) -> pipeline132/1(d)
  pipeline120/1(q) -> ab_re0/1(addr)
  cmult2/1(ab) -> c_to_ri2/1
  simple_bram_vacc17/2(valid) -> pipeline138/1(d)
  pipeline133/1(q) -> ab_re1/1(addr)
  pipeline145/1(q) -> simple_bram_vacc17/1(new_acc)
  Delay62/1 -> pipeline168/1(d)
  pipeline144/1(q) -> pipeline128/1(d)
  pipeline138/1(q) -> ab_re0/3(we)
  simple_bram_vacc17/1(dout) -> Reinterpret23/1
  pipeline155/1(q) -> ab_re3/3(we)
  pipeline154/1(q) -> ab_re3/2(data_in)
  simple_bram_vacc18/1(dout) -> Reinterpret24/1
  pipeline163/1(q) -> ab_re2/2(data_in)
  pipeline156/1(q) -> ab_re3/1(addr)
  pipeline136/1(q) -> ab_im1/3(we)
  simple_bram_vacc23/1(dout) -> Reinterpret25/1
  pipeline217/1(q) -> pipeline214/1(d)
  pipeline203/1(q) -> ab_im6/2(data_in)
  Reinterpret32/1 -> pipeline180/1(d)
  simple_bram_vacc29/1(dout) -> Reinterpret35/1
  pipeline206/1(q) -> ab_re7/3(we)
  Delay73/1 -> pipeline217/1(d)
  pipeline185/1(q) -> ab_im5/2(data_in)
  Delay68/1 -> pipeline192/1(d)
  simple_bram_vacc25/1(dout) -> Reinterpret31/1
  simple_bram_vacc28/1(dout) -> Reinterpret30/1
  cmult7/1(ab) -> c_to_ri7/1
  Reinterpret29/1 -> pipeline182/1(d)
  ab_re4/1(data_out) -> Terminator38/1
  ab_im6/1(data_out) -> Terminator43/1
  ab_re5/1(data_out) -> Terminator40/1
  ab_re6/1(data_out) -> Terminator42/1
  pipeline208/1(q) -> ab_im7/2(data_in)
  simple_bram_vacc25/2(valid) -> pipeline189/1(d)
  pipeline180/1(q) -> ab_im4/2(data_in)
  ab_im7/1(data_out) -> Terminator45/1
  Reinterpret33/1 -> pipeline205/1(d)
  ab_im4/1(data_out) -> Terminator39/1
  Delay74/1 -> pipeline218/1(d)
  pipeline183/1(q) -> ab_re5/3(we)
  Reinterpret35/1 -> pipeline211/1(d)
  pipeline216/1(q) -> pipeline200/1(d)
  pipeline182/1(q) -> ab_re5/2(data_in)
  pipeline207/1(q) -> ab_re7/1(addr)
  simple_bram_vacc31/2(valid) -> pipeline206/1(d)
  cmult4/1(ab) -> c_to_ri4/1
  pipeline211/1(q) -> ab_re6/2(data_in)
  pipeline181/1(q) -> ab_im4/3(we)
  ab_im5/1(data_out) -> Terminator41/1
  simple_bram_vacc32/2(valid) -> pipeline210/1(d)
  Delay67/1 -> pipeline215/1(d)
  Reinterpret30/1 -> pipeline185/1(d)
  pipeline173/1(q) -> ab_im6/1(addr)
  simple_bram_vacc30/1(dout) -> Reinterpret36/1
  simple_bram_vacc32/1(dout) -> Reinterpret34/1
  cmult5/1(ab) -> c_to_ri5/1
  simple_bram_vacc28/2(valid) -> pipeline187/1(d)
  pipeline195/1(q) -> pipeline179/1(d)
  pipeline188/1(q) -> ab_re4/2(data_in)
  pipeline189/1(q) -> ab_re4/3(we)
  simple_bram_vacc29/2(valid) -> pipeline212/1(d)
  pipeline190/1(q) -> ab_im5/1(addr)
  simple_bram_vacc27/1(dout) -> Reinterpret29/1
  pipeline210/1(q) -> ab_im7/3(we)
  pipeline205/1(q) -> ab_re7/2(data_in)
  Delay72/1 -> pipeline195/1(d)
  simple_bram_vacc26/1(dout) -> Reinterpret32/1
  ab_re7/1(data_out) -> Terminator44/1
  pipeline187/1(q) -> ab_im5/3(we)
  simple_bram_vacc31/1(dout) -> Reinterpret33/1
  pipeline198/1(q) -> ab_im4/1(addr)
  pipeline175/1(q) -> ab_re6/1(addr)
  pipeline192/1(q) -> pipeline197/1(d)
  pipeline193/1(q) -> pipeline177/1(d)
  pipeline194/1(q) -> pipeline191/1(d)
  pipeline215/1(q) -> pipeline172/1(d)
  Reinterpret36/1 -> pipeline203/1(d)
  Delay69/1 -> pipeline193/1(d)
  pipeline212/1(q) -> ab_re6/3(we)
  simple_bram_vacc30/2(valid) -> pipeline204/1(d)
  simple_bram_vacc26/2(valid) -> pipeline181/1(d)
  Reinterpret34/1 -> pipeline208/1(d)
  simple_bram_vacc27/2(valid) -> pipeline183/1(d)
  pipeline174/1(q) -> ab_re4/1(addr)
  cmult6/1(ab) -> c_to_ri6/1
  pipeline184/1(q) -> ab_re5/1(addr)
  Delay70/1 -> pipeline216/1(d)
  pipeline213/1(q) -> ab_im7/1(addr)
  Reinterpret31/1 -> pipeline188/1(d)
  Delay71/1 -> pipeline194/1(d)
  pipeline204/1(q) -> ab_im6/3(we)
  pipeline218/1(q) -> pipeline202/1(d)
  c_to_ri/1 -> Delay76/1
  Delay76/1 -> Delay91/1
  c_to_ri/2 -> Delay77/1
  Delay77/1 -> Delay92/1
  Delay80/1 -> Delay94/1
  c_to_ri1/2 -> Delay80/1
  Delay79/1 -> Delay93/1
  c_to_ri1/1 -> Delay79/1
  c_to_ri3/1 -> Delay81/1
  Delay81/1 -> Delay97/1
  c_to_ri3/2 -> Delay82/1
  Delay82/1 -> Delay98/1
  Delay78/1 -> Delay96/1
  c_to_ri2/2 -> Delay78/1
  Delay75/1 -> Delay95/1
  c_to_ri2/1 -> Delay75/1
  c_to_ri6/1 -> Delay83/1
  Delay83/1 -> Delay103/1
  c_to_ri6/2 -> Delay86/1
  Delay86/1 -> Delay104/1
  Delay90/1 -> Delay106/1
  c_to_ri7/2 -> Delay90/1
  Delay89/1 -> Delay105/1
  c_to_ri7/1 -> Delay89/1
  c_to_ri5/1 -> Delay87/1
  Delay87/1 -> Delay101/1
  c_to_ri5/2 -> Delay88/1
  Delay88/1 -> Delay102/1
  Delay85/1 -> Delay100/1
  c_to_ri4/2 -> Delay85/1
  Delay84/1 -> Delay99/1
  c_to_ri4/1 -> Delay84/1
  Delay91/1 -> Delay60/1
  Delay92/1 -> Delay61/1
  Delay93/1 -> Delay63/1
  Delay94/1 -> Delay64/1
  Delay95/1 -> Delay59/1
  Delay96/1 -> Delay62/1
  Delay97/1 -> Delay65/1
  Delay98/1 -> Delay66/1
  Delay104/1 -> Delay70/1
  Delay101/1 -> Delay71/1
  Delay105/1 -> Delay73/1
  Delay100/1 -> Delay69/1
  Delay102/1 -> Delay72/1
  Delay103/1 -> Delay67/1
  Delay99/1 -> Delay68/1
  Delay106/1 -> Delay74/1
  pipeline201/1(q) -> simple_bram_vacc32/1(new_acc)
  pipeline202/1(q) -> simple_bram_vacc32/2(din)
  pipeline123/1(q) -> simple_bram_vacc18/1(new_acc)
  pipeline124/1(q) -> simple_bram_vacc18/2(din)
  pipeline135/1(q) -> simple_bram_vacc19/1(new_acc)
  pipeline140/1(q) -> simple_bram_vacc19/2(din)
  pipeline127/1(q) -> simple_bram_vacc20/1(new_acc)
  pipeline128/1(q) -> simple_bram_vacc20/2(din)
  pipeline115/1(q) -> simple_bram_vacc21/1(new_acc)
  pipeline116/1(q) -> simple_bram_vacc21/2(din)
  pipeline148/1(q) -> simple_bram_vacc22/1(new_acc)
  pipeline149/1(q) -> simple_bram_vacc22/2(din)
  pipeline159/1(q) -> simple_bram_vacc23/1(new_acc)
  pipeline166/1(q) -> simple_bram_vacc23/2(din)
  pipeline150/1(q) -> simple_bram_vacc24/1(new_acc)
  pipeline151/1(q) -> simple_bram_vacc24/2(din)
  pipeline196/1(q) -> simple_bram_vacc25/1(new_acc)
  pipeline197/1(q) -> simple_bram_vacc25/2(din)
  pipeline176/1(q) -> simple_bram_vacc26/1(new_acc)
  pipeline177/1(q) -> simple_bram_vacc26/2(din)
  pipeline186/1(q) -> simple_bram_vacc27/1(new_acc)
  pipeline191/1(q) -> simple_bram_vacc27/2(din)
  pipeline178/1(q) -> simple_bram_vacc28/1(new_acc)
  pipeline179/1(q) -> simple_bram_vacc28/2(din)
  pipeline171/1(q) -> simple_bram_vacc29/1(new_acc)
  pipeline209/1(q) -> simple_bram_vacc31/1(new_acc)
  pipeline199/1(q) -> simple_bram_vacc30/1(new_acc)
  pipeline172/1(q) -> simple_bram_vacc29/2(din)
  pipeline200/1(q) -> simple_bram_vacc30/2(din)
  pipeline214/1(q) -> simple_bram_vacc31/2(din)
  Constant16/1 -> acc_len/1(sim_1)
  pipeline435/1(q) -> [cnt_rst]
  Constant19/1 -> cnt_rst/1(sim_1)
  cnt_rst/1(in_reg) -> pipeline435/1(d)
  acc_len/1(in_reg) -> pipeline9/1(d)
  pipeline9/1(q) -> [acc_len]
  acc_cnt/1(sim_out_reg) -> Terminator81/1
  Delay214/1 -> acc_cntr/2
  [cnt_rst] -> acc_cntr/1
  acc_cntrl/1(new_acc) -> Delay214/1, pipeline113/1(d), pipeline103/1(d), pipeline145/1(d), pipeline123/1(d), pipeline135/1(d), pipeline127/1(d), pipeline115/1(d), pipeline159/1(d), pipeline150/1(d), pipeline176/1(d), pipeline186/1(d), pipeline178/1(d), pipeline171/1(d), pipeline199/1(d), pipeline209/1(d), pipeline201/1(d), pipeline196/1(d), pipeline148/1(d), pipeline110/1(d), pipeline93/1(d), pipeline80/1(d), pipeline86/1(d), pipeline68/1(d), pipeline112/1(d), pipeline62/1(d), pipeline46/1(d), pipeline51/1(d), pipeline37/1(d), pipeline25/1(d), pipeline30/1(d), pipeline16/1(d), pipeline6/1(d), pipeline125/1(d)
  Delay213/1 -> acc_cnt/1(out_reg)
  acc_cntr/1 -> Delay213/1
  Delay48/1 -> pipeline8/1(d)
  sync_gen/1(sync_out) -> Delay48/1
  pipeline8/1(q) -> [sync_gen]
